simulink model "snap2_f_200msps_64i_4096c_for_8_11_2124fpg"  (R2019a)
settings: Solver=VariableStepDiscrete  StartTime=0.0  StopTime=10.0  RelTol=1e-3
undefined symbols (not defined in the file): datetime, posixtime
libraries: xbsIndex_r4 x17164, casper_library_misc x251, xps_library x14, casper_library_delays x12, casper_library_sources x4
(part 12 of 196)

== system snap2_f_200msps_64i_4096c_for_8_11_2124fpg/cosmic_ray/buffer_and_packetizer ==
  note: should always be zero
  note: Counter number of clock cycles that the board spends in readout state during one readout.
  note: Enable the timer while the board is in readout_state, reset from software.
  note: 4 delays:
      | 1 for OR before buffer address counter
      | 1 for address counter to start after enabled
      | 1 for buffer
      | 1 for block sel
  note: note: buffer address increments 2 clock cycles after read_word
  note: This controls the wait between packets.
  In1 : Inport
  In2 : Inport  Port=2
  In3 : Inport  Port=3
  In4 : Inport  Port=4
  In5 : Inport  Port=5
  In6 : Inport  Port=6
  In7 : Inport  Port=7
  In8 : Inport  Port=8
  In9 : Inport  Port=9
  AddSub : xbsIndex_r4/AddSub  mode=Addition  use_carryin=off  use_carryout=off  en=off  latency=1  precision=Full  arith_type=Unsigned  n_bits=16  bin_pt=14  quantization=Truncate  overflow=Wrap  use_behavioral_HDL=off  hw_selection=Fabric  pipelined=off  dbl_ovrd=off  block_type=addsub
  Constant22 : xbsIndex_r4/Constant  const=0  gui_display_data_type=Boolean  arith_type=Boolean  n_bits=16  bin_pt=14  preci_type=Single  exp_width=8  frac_width=24  explicit_period=on  period=1  equ=P=C  opselect=C  inp2=PCIN>>17  opr=+  inp1=P  carry=CIN  dbl_ovrd=off  block_type=constant
  Constant23 : xbsIndex_r4/Constant  const=0  gui_display_data_type=Boolean  arith_type=Boolean  n_bits=16  bin_pt=14  preci_type=Single  exp_width=8  frac_width=24  explicit_period=on  period=1  equ=P=C  opselect=C  inp2=PCIN>>17  opr=+  inp1=P  carry=CIN  dbl_ovrd=off  block_type=constant
  Constant25 : xbsIndex_r4/Constant  const=3  gui_display_data_type=Fixed-point  arith_type=Unsigned  n_bits=32  bin_pt=0  preci_type=Single  exp_width=8  frac_width=24  explicit_period=on  period=1  equ=P=C  opselect=C  inp2=PCIN>>17  opr=+  inp1=P  carry=CIN  dbl_ovrd=off  block_type=constant
  Constant26 : xbsIndex_r4/Constant  const=2  gui_display_data_type=Fixed-point  arith_type=Unsigned  n_bits=32  bin_pt=0  preci_type=Single  exp_width=8  frac_width=24  explicit_period=on  period=1  equ=P=C  opselect=C  inp2=PCIN>>17  opr=+  inp1=P  carry=CIN  dbl_ovrd=off  block_type=constant
  Constant28 : xbsIndex_r4/Constant  const=0  gui_display_data_type=Boolean  arith_type=Boolean  n_bits=16  bin_pt=14  preci_type=Single  exp_width=8  frac_width=24  explicit_period=on  period=1  equ=P=C  opselect=C  inp2=PCIN>>17  opr=+  inp1=P  carry=CIN  dbl_ovrd=off  block_type=constant
  Constant29 : xbsIndex_r4/Constant  const=1  gui_display_data_type=Fixed-point  arith_type=Unsigned  n_bits=32  bin_pt=0  preci_type=Single  exp_width=8  frac_width=24  explicit_period=on  period=1  equ=P=C  opselect=C  inp2=PCIN>>17  opr=+  inp1=P  carry=CIN  dbl_ovrd=off  block_type=constant
  Constant30 : xbsIndex_r4/Constant  const=0  gui_display_data_type=Fixed-point  arith_type=Unsigned  n_bits=32  bin_pt=0  preci_type=Single  exp_width=8  frac_width=24  explicit_period=on  period=1  equ=P=C  opselect=C  inp2=PCIN>>17  opr=+  inp1=P  carry=CIN  dbl_ovrd=off  block_type=constant
  Constant33 : xbsIndex_r4/Constant  const=256  gui_display_data_type=Fixed-point  arith_type=Unsigned  n_bits=9  bin_pt=0  preci_type=Single  exp_width=8  frac_width=24  explicit_period=on  period=1  equ=P=C  opselect=C  inp2=PCIN>>17  opr=+  inp1=P  carry=CIN  dbl_ovrd=off  block_type=constant
  Counter2 : xbsIndex_r4/Counter  cnt_type=Free Running  cnt_to=Inf  operation=Up  start_count=0  cnt_by_val=1  arith_type=Unsigned  n_bits=32  bin_pt=0  load_pin=off  rst=off  en=on  explicit_period=on  period=1  use_behavioral_HDL=off  implementation=Fabric  dbl_ovrd=off  block_type=counter
  Counter5 : xbsIndex_r4/Counter  cnt_type=Free Running  cnt_to=Inf  operation=Up  start_count=0  cnt_by_val=1  arith_type=Unsigned  n_bits=32  bin_pt=0  load_pin=off  rst=off  en=on  explicit_period=on  period=1  use_behavioral_HDL=off  implementation=Fabric  dbl_ovrd=off  block_type=counter
  Delay1 : xbsIndex_r4/Delay  rst=off  infoeditControl=Selection of Reset will increase slice count due to use of real FFs and instead of SRLs  en=off  latency=2  reg_retiming=off  dbl_ovrd=off  block_type=delay
  Delay{2,4..6,10} (5 alike) : xbsIndex_r4/Delay  rst=off  infoeditControl=Selection of Reset will increase slice count due to use of real FFs and instead of SRLs  en=off  latency=1  reg_retiming=on  dbl_ovrd=off  block_type=delay
  Delay3 : xbsIndex_r4/Delay  rst=off  infoeditControl=Selection of Reset will increase slice count due to use of real FFs and instead of SRLs  en=off  latency=1  reg_retiming=off  dbl_ovrd=off  block_type=delay
  Delay7 : xbsIndex_r4/Delay  rst=off  infoeditControl=Selection of Reset will increase slice count due to use of real FFs and instead of SRLs  en=off  latency=4  reg_retiming=off  dbl_ovrd=off  block_type=delay
  Delay8 : xbsIndex_r4/Delay  rst=off  infoeditControl=Selection of Reset will increase slice count due to use of real FFs and instead of SRLs  en=off  latency=4  reg_retiming=off  dbl_ovrd=off  block_type=delay
  Delay9 : xbsIndex_r4/Delay  rst=off  infoeditControl=Selection of Reset will increase slice count due to use of real FFs and instead of SRLs  en=off  latency=2  reg_retiming=off  dbl_ovrd=off  block_type=delay
  Inverter : xbsIndex_r4/Inverter  en=off  latency=1  dbl_ovrd=off  block_type=inv
  Logical3 : xbsIndex_r4/Logical  logical_function=AND  inputs=2  en=off  latency=1  precision=Full  arith_type=Unsigned  n_bits=16  bin_pt=0  align_bp=on  dbl_ovrd=off  block_type=logical
  Logical4 : xbsIndex_r4/Logical  logical_function=AND  inputs=2  en=off  latency=1  precision=Full  arith_type=Unsigned  n_bits=16  bin_pt=0  align_bp=on  dbl_ovrd=off  block_type=logical
  Logical7 : xbsIndex_r4/Logical  logical_function=OR  inputs=2  en=off  latency=1  precision=Full  arith_type=Unsigned  n_bits=16  bin_pt=0  align_bp=on  dbl_ovrd=off  block_type=logical
  Mux1 : xbsIndex_r4/Mux  inputs=4  en=off  latency=1  precision=Full  arith_type=Unsigned  n_bits=16  bin_pt=14  quantization=Truncate  overflow=Wrap  dbl_ovrd=off  block_type=mux
  Relational2 : xbsIndex_r4/Relational  mode=a>b  op_type=Bool  en=off  latency=1  dbl_ovrd=off  block_type=relational
  Scope{3..5,7..15,18} (13 alike) : Scope
  Single Port RAM{,1..4} (5 alike) : xbsIndex_r4/Single Port RAM  depth=4096  initVector=sin(pi*(0:15)/16)  distributed_mem=Block RAM  write_mode=Read After Write  rst=off  init_reg=0  en=off  latency=1  optimize=Power  dbl_ovrd=off  block_type=spram
  Slice2 : xbsIndex_r4/Slice  nbits=1  boolean_output=on  mode=Upper Bit Location + Width  bit1=0  base1=MSB of Input  bit0=0  base0=LSB of Input  dbl_ovrd=off  block_type=slice
  Slice3 : xbsIndex_r4/Slice  nbits=1  boolean_output=on  mode=Upper Bit Location + Width  bit1=0  base1=MSB of Input  bit0=0  base0=LSB of Input  dbl_ovrd=off  block_type=slice
  Terminator21 : Terminator
  block_counter : xbsIndex_r4/Counter  cnt_type=Free Running  cnt_to=Inf  operation=Up  start_count=0  cnt_by_val=1  arith_type=Unsigned  n_bits=2  bin_pt=0  load_pin=off  rst=off  en=on  explicit_period=on  period=1  use_behavioral_HDL=off  implementation=Fabric  dbl_ovrd=off  block_type=counter
  buffer_addr : xbsIndex_r4/Counter  cnt_type=Free Running  cnt_to=Inf  operation=Up  start_count=0  cnt_by_val=1  arith_type=Unsigned  n_bits=12  bin_pt=0  load_pin=off  rst=off  en=on  explicit_period=on  period=1  use_behavioral_HDL=off  implementation=Fabric  dbl_ovrd=off  block_type=counter
  bus_create : Subsystem  AncestorBlock=casper_library_flow_control/bus_create  mask(bus_create){inputNum=3}
      mask prompts: inputNum "Number of inputs:"
      mask init:
      | bus_create_init(gcb,...
      |     'inputNum', inputNum);
  bus_create1 : Subsystem  AncestorBlock=casper_library_flow_control/bus_create  mask(bus_create){inputNum=3}
      mask prompts: inputNum "Number of inputs:"
      mask init:
      | bus_create_init(gcb,...
      |     'inputNum', inputNum);
  bus_create2 : Subsystem  AncestorBlock=casper_library_flow_control/bus_create  mask(bus_create){inputNum=3}
      mask prompts: inputNum "Number of inputs:"
      mask init:
      | bus_create_init(gcb,...
      |     'inputNum', inputNum);
  bus_create3 : Subsystem  AncestorBlock=casper_library_flow_control/bus_create  mask(bus_create){inputNum=3}
      mask prompts: inputNum "Number of inputs:"
      mask init:
      | bus_create_init(gcb,...
      |     'inputNum', inputNum);
  bus_expand : Subsystem  AncestorBlock=casper_library_flow_control/bus_expand  mask(bus_expand){mode=divisions of equal size, outputNum=4, outputWidth=160, outputBinaryPt=0, outputArithmeticType=0, show_format=off, outputToWorkspace=off, variablePrefix=out, outputToModelAsWell=on}
      mask prompts: outputNum "Number of outputs:"; outputWidth "Output width:"; outputBinaryPt "Output binary point position:"; outputArithmeticType "Output arithmetic type (ufix=0, fix=1, bool=2):"; show_format "Print format string?"; outputToWorkspace "Output to workspace?"; variablePrefix "Variable name prefix:"; outputToModelAsWell "Output to model as well?"
      mask help:
      | Expands a 'bus' made using a bus_create or Xilinx
      | concat block.
      | The input will be divided into the specified number
      | of outputs with the specified arithmetic types.
      | Divisions are made from most significant bit.
      mask init: same as snap2_f_200msps_64i_4096c_for_8_11_2124fpg/autocorr/bus_expand
      settings: AttributesFormatString=4 outputs
      mask TypeOptions mode: divisions of equal size | divisions of arbitrary size
      mask Callback mode: bus_expand_callback();
      mask Callback outputWidth: bus_expand_callback();
      mask Callback outputToWorkspace: bus_expand_callback();
  count-limited-counter : Subsystem
  edge_detect11 : Subsystem  AncestorBlock=casper_library_misc/edge_detect  mask(edge_detect){edge=Falling, polarity=Active High, x_in=[0 0.08 0.08 0.16 0.16 0.24 0.24 0.32 0.32 0.4], y_in=[-1 -1  1  1  1  1  1  1  1  1], x_out=[0.6 0.68 0.68 0.76 0.76 0.84 0.84 0.92 0.92 1], y_out=[-1 -1  1  1 -1 -1 -1 -1 -1 -1]}
      mask prompts: edge "Edge Type"; polarity "Output Polarity"; x_in "Input X Positions"; y_in "Input Y Positions"; x_out "Output X Positions"; y_out "Output Y Positions"
      mask help: Output is active for one-cycle after each edge of the specified type (i.e. rising, falling or both).
      mask init: same as snap2_f_200msps_64i_4096c_for_8_11_2124fpg/pulse_ext/posedge
      settings: AttributesFormatString=|
      | falling edges
      | active high
      mask Help: Output is active for one-cycle after each edge of the specified type (i.e. rising, falling, or both).  Latency is zero.
      mask TypeOptions edge: Rising | Falling | Both
      mask TypeOptions polarity: Active High | Active Low
      mask Display:
      | plot(x_in_num,0.5+y_in_num*0.2);
      | plot(x_out_num,0.5+y_out_num*0.2);
  edge_detect12 : Subsystem  AncestorBlock=casper_library_misc/edge_detect  mask(edge_detect){edge=Falling, polarity=Active High, x_in=[0 0.08 0.08 0.16 0.16 0.24 0.24 0.32 0.32 0.4], y_in=[-1 -1  1  1  1  1  1  1  1  1], x_out=[0.6 0.68 0.68 0.76 0.76 0.84 0.84 0.92 0.92 1], y_out=[-1 -1  1  1 -1 -1 -1 -1 -1 -1]}
      mask prompts: edge "Edge Type"; polarity "Output Polarity"; x_in "Input X Positions"; y_in "Input Y Positions"; x_out "Output X Positions"; y_out "Output Y Positions"
      mask help: Output is active for one-cycle after each edge of the specified type (i.e. rising, falling or both).
      mask init: same as snap2_f_200msps_64i_4096c_for_8_11_2124fpg/pulse_ext/posedge
      settings: AttributesFormatString=|
      | falling edges
      | active high
      mask Help: Output is active for one-cycle after each edge of the specified type (i.e. rising, falling, or both).  Latency is zero.
      mask TypeOptions edge: Rising | Falling | Both
      mask TypeOptions polarity: Active High | Active Low
      mask Display:
      | plot(x_in_num,0.5+y_in_num*0.2);
      | plot(x_out_num,0.5+y_out_num*0.2);
  edge_detect13 : Subsystem  AncestorBlock=casper_library_misc/edge_detect  mask(edge_detect){edge=Falling, polarity=Active High, x_in=[0 0.08 0.08 0.16 0.16 0.24 0.24 0.32 0.32 0.4], y_in=[-1 -1  1  1  1  1  1  1  1  1], x_out=[0.6 0.68 0.68 0.76 0.76 0.84 0.84 0.92 0.92 1], y_out=[-1 -1  1  1 -1 -1 -1 -1 -1 -1]}
      mask prompts: edge "Edge Type"; polarity "Output Polarity"; x_in "Input X Positions"; y_in "Input Y Positions"; x_out "Output X Positions"; y_out "Output Y Positions"
      mask help: Output is active for one-cycle after each edge of the specified type (i.e. rising, falling or both).
      mask init: same as snap2_f_200msps_64i_4096c_for_8_11_2124fpg/pulse_ext/posedge
      settings: AttributesFormatString=|
      | falling edges
      | active high
      mask Help: Output is active for one-cycle after each edge of the specified type (i.e. rising, falling, or both).  Latency is zero.
      mask TypeOptions edge: Rising | Falling | Both
      mask TypeOptions polarity: Active High | Active Low
      mask Display:
      | plot(x_in_num,0.5+y_in_num*0.2);
      | plot(x_out_num,0.5+y_out_num*0.2);
  edge_detect15 : casper_library_misc/edge_detect  edge=Rising  polarity=Active High  x_in=[0 0.08 0.08 0.16 0.16 0.24 0.24 0.32 0.32 0.4]  y_in="[-1 -1  1  1  1  1  1  1  1  1]"  x_out=[0.6 0.68 0.68 0.76 0.76 0.84 0.84 0.92 0.92 1]  y_out="[-1 -1  1  1 -1 -1 -1 -1 -1 -1]"
  packet_counter : xbsIndex_r4/Counter  cnt_type=Free Running  cnt_to=Inf  operation=Up  start_count=0  cnt_by_val=1  arith_type=Unsigned  n_bits=4  bin_pt=0  load_pin=off  rst=off  en=on  explicit_period=on  period=1  use_behavioral_HDL=off  implementation=Fabric  dbl_ovrd=off  block_type=counter
  readout_timer1 : xbsIndex_r4/Counter  cnt_type=Free Running  cnt_to=1  operation=Up  start_count=0  cnt_by_val=1  arith_type=Unsigned  n_bits=32  bin_pt=0  load_pin=off  rst=on  en=on  explicit_period=off  period=1  use_behavioral_HDL=off  implementation=Fabric  dbl_ovrd=off  block_type=counter
  timestamp : xbsIndex_r4/Counter  cnt_type=Free Running  cnt_to=Inf  operation=Up  start_count=0  cnt_by_val=1  arith_type=Unsigned  n_bits=64  bin_pt=0  load_pin=off  rst=on  en=off  explicit_period=on  period=1  use_behavioral_HDL=on  implementation=Fabric  dbl_ovrd=off  block_type=counter
  Out1 : Outport
  Out2 : Outport  Port=2
  Out3 : Outport  Port=3
  Out4 : Outport  Port=4
  Out5 : Outport  Port=5
  Out6 : Outport  Port=6
  Out7 : Outport  Port=7
  Out8 : Outport  Port=8
  Out9 : Outport  Port=9
  Out10 : Outport  Port=10
  Out11 : Outport  Port=11
  Out12 : Outport  Port=12
  Out13 : Outport  Port=13
  timestamp_out : Outport  Port=14
  wires:
  [cr_eof] -> packet_counter/1
  bus_create/1(bus_out) -> Mux1/5
  bus_expand/3(out2) -> Single Port RAM2/2
  timestamp/1 -> Delay2/1, timestamp_out/1
  Logical3/1 -> Counter5/1
  Constant30/1 -> bus_create3/2(in2)
  AddSub/1 -> count-limited-counter/2(limit)
  [read_word] -> Scope3/1
  [cr_40g_dest_ip] -> Out4/1
  Slice3/1 -> edge_detect13/1(in)
  [cr_eof] -> Scope4/1
  [increment_block] -> block_counter/1
  Delay9/1 -> [buffer_we]
  In1/1 -> [packetwait]
  Relational2/1 -> Delay3/1, edge_detect11/1(in)
  Slice2/1 -> edge_detect12/1(in)
  [buffer_we] -> Logical4/2, Scope11/1
  [readout_state] -> Scope8/1
  Constant22/1 -> Out8/1
  bus_expand/4(lsb_out1) -> Single Port RAM3/2
  edge_detect12/1(out) -> [increment_block]
  [buffer_we] -> Single Port RAM1/3
  [packet_subset_count] -> Scope14/1
  Inverter/1 -> [addr_ctr_stop], Delay9/1
  bus_create2/1(bus_out) -> Mux1/3
  Single Port RAM/1 -> bus_create3/1(in1)
  Single Port RAM4/1 -> bus_create2/3(in3), bus_create3/3(in3), bus_create1/3(in3), bus_create/3(in3)
  Delay8/1 -> Out3/1
  [cr_40g_dest_port] -> Out5/1
  Constant23/1 -> Out7/1
  Constant28/1 -> Out9/1
  Logical7/1 -> buffer_addr/1
  [buffer_we] -> Single Port RAM4/3
  In2/1 -> [timestamp_ctr_rst]
  In3/1 -> [packetizer_sync]
  [read_word] -> Logical4/1, Scope5/1
  [increment_block] -> Scope9/1
  packet_counter/1 -> Slice2/1, [packet_subset_count]
  readout_timer1/1 -> Out12/1, Scope15/1
  In5/1 -> [cr_40g_dest_ip]
  [readout_state] -> count-limited-counter/1(en)
  Counter2/1 -> Out10/1
  Mux1/1 -> Out2/1
  bus_expand/1(msb_out4) -> Single Port RAM/2
  [timestamp_ctr_rst] -> timestamp/1
  In4/1 -> [cr_40g_rst]
  [read_word] -> Logical3/2
  In6/1 -> [cr_40g_dest_port]
  In8/1 -> bus_expand/1(bus_in)
  edge_detect15/1 -> readout_timer1/1
  [readout_timer_rst] -> edge_detect15/1
  [packetizer_sync] -> Delay10/1
  [buffer_we] -> Single Port RAM2/3
  bus_create3/1(bus_out) -> Mux1/2
  [cr_40g_rst] -> Out1/1
  Delay7/1 -> Out6/1
  Single Port RAM3/1 -> bus_create/1(in1)
  Logical4/1 -> Scope7/1
  [cr_eof] -> Counter2/1
  [packetwait] -> AddSub/2, Relational2/2
  Single Port RAM2/1 -> bus_create1/1(in1)
  In7/1 -> [readout_timer_rst]
  [addr_ctr_stop] -> Logical7/2
  Constant26/1 -> bus_create1/2(in2)
  [cr_eof] -> Logical3/1
  [buffer_addr] -> Single Port RAM/1
  Constant29/1 -> bus_create2/2(in2)
  [buffer_addr] -> Single Port RAM1/1
  [buffer_we] -> Single Port RAM/3
  Constant33/1 -> AddSub/1
  edge_detect13/1(out) -> [readout_done]
  [cr_eof] -> Delay7/1
  bus_expand/2(out3) -> Single Port RAM1/2
  Delay10/1 -> Delay4/1
  [block_step] -> Scope13/1
  [buffer_addr] -> Single Port RAM4/1
  Delay6/1 -> Terminator21/1
  count-limited-counter/1(out) -> Scope18/1, Relational2/1
  Delay1/1 -> Mux1/1
  Single Port RAM1/1 -> bus_create2/1(in1)
  [readout_state] -> readout_timer1/2
  [buffer_addr] -> Single Port RAM3/1
  [buffer_addr] -> Single Port RAM2/1
  edge_detect11/1(out) -> [cr_eof]
  [block_step] -> Delay1/1
  block_counter/1 -> [block_step], Slice3/1
  [readout_state] -> Inverter/1
  buffer_addr/1 -> [buffer_addr]
  Delay5/1 -> Delay6/1
  Constant25/1 -> bus_create/2(in2)
  Delay3/1 -> [read_word]
  [buffer_we] -> Single Port RAM3/3
  Counter5/1 -> Out11/1
  Delay2/1 -> Single Port RAM4/2
  [read_word] -> Logical7/1
  [buffer_addr] -> Scope12/1
  Delay4/1 -> Delay5/1
  [read_word] -> Delay8/1
  [readout_done] -> Scope10/1
  bus_create1/1(bus_out) -> Mux1/4
  In9/1 -> [readout_state]
  [readout_done] -> Out13/1

== system snap2_f_200msps_64i_4096c_for_8_11_2124fpg/cosmic_ray/bus_create4 ==
  in1 : Inport
  in2 : Inport  Port=2
  concatenate : xbsIndex_r4/Concat  num_inputs=2  dbl_ovrd=off  block_type=concat
  reinterpret1 : xbsIndex_r4/Reinterpret  force_arith_type=on  arith_type=Unsigned  force_bin_pt=on  bin_pt=0  block_type=reinterpret
      settings: infoedit="Changes signal type without altering the binary representation.   You can changed the signal between signed and unsigned, and relocate the binary point.<br><br>Hardware notes: In hardware this block costs nothing.<br><br>Example:  Suppose the input is 6 bits wide, signed, with 2 fractional bits, and the output is forced to unsigned with 0 fractional bits.  Then an input of -2.0 (1110.00 in binary 2's complement) becomes an output of 56 (111000 in binary)."
  reinterpret2 : xbsIndex_r4/Reinterpret  force_arith_type=on  arith_type=Unsigned  force_bin_pt=on  bin_pt=0  block_type=reinterpret
      settings: infoedit="Changes signal type without altering the binary representation.   You can changed the signal between signed and unsigned, and relocate the binary point.<br><br>Hardware notes: In hardware this block costs nothing.<br><br>Example:  Suppose the input is 6 bits wide, signed, with 2 fractional bits, and the output is forced to unsigned with 0 fractional bits.  Then an input of -2.0 (1110.00 in binary 2's complement) becomes an output of 56 (111000 in binary)."
  bus_out : Outport
  wires:
  concatenate/1 -> bus_out/1
  in1/1 -> reinterpret1/1
  reinterpret1/1 -> concatenate/1
  in2/1 -> reinterpret2/1
  reinterpret2/1 -> concatenate/2

== system snap2_f_200msps_64i_4096c_for_8_11_2124fpg/cosmic_ray/bus_create5 ==
  in1 : Inport
  in2 : Inport  Port=2
  concatenate : xbsIndex_r4/Concat  num_inputs=2  dbl_ovrd=off  block_type=concat
  reinterpret1 : xbsIndex_r4/Reinterpret  force_arith_type=on  arith_type=Unsigned  force_bin_pt=on  bin_pt=0  block_type=reinterpret
      settings: infoedit="Changes signal type without altering the binary representation.   You can changed the signal between signed and unsigned, and relocate the binary point.<br><br>Hardware notes: In hardware this block costs nothing.<br><br>Example:  Suppose the input is 6 bits wide, signed, with 2 fractional bits, and the output is forced to unsigned with 0 fractional bits.  Then an input of -2.0 (1110.00 in binary 2's complement) becomes an output of 56 (111000 in binary)."
  reinterpret2 : xbsIndex_r4/Reinterpret  force_arith_type=on  arith_type=Unsigned  force_bin_pt=on  bin_pt=0  block_type=reinterpret
      settings: infoedit="Changes signal type without altering the binary representation.   You can changed the signal between signed and unsigned, and relocate the binary point.<br><br>Hardware notes: In hardware this block costs nothing.<br><br>Example:  Suppose the input is 6 bits wide, signed, with 2 fractional bits, and the output is forced to unsigned with 0 fractional bits.  Then an input of -2.0 (1110.00 in binary 2's complement) becomes an output of 56 (111000 in binary)."
  bus_out : Outport
  wires:
  reinterpret2/1 -> concatenate/2
  in2/1 -> reinterpret2/1
  reinterpret1/1 -> concatenate/1
  in1/1 -> reinterpret1/1
  concatenate/1 -> bus_out/1

== system snap2_f_200msps_64i_4096c_for_8_11_2124fpg/cosmic_ray/coincidence_trigger ==
  note: FIR here?
  note: FIR here?
  note: The ant_threshold block should output a 64 bit signal which is 64 boolean signals bussed together, each indicating whether or not the signal on that antenna exceeded the threshold.
  note: The select_trigger_antennas block sets to zero all streams which are not participating in the core trigger. The select_vetos block sets to zero all antenna streams which are not veto antennas.
  note: Extend pulse for time to wait between trigger and veto.
  note: FIR here?
  note: Determine whether there has been a trigger and not a veto.
  note: FIR here?
  note: Square each voltage stream by copying the bussed voltages and multiplying together, elementwise.
  note: Trigger coincidencer and veto coincidencer are the same block with a different window length.
  In1 : Inport
  In2 : Inport  Port=2
  In3 : Inport  Port=3
  In4 : Inport  Port=4
  In5 : Inport  Port=5
  In6 : Inport  Port=6
  In7 : Inport  Port=7
  In8 : Inport  Port=8
  In9 : Inport  Port=9
  In10 : Inport  Port=10
  AddSub1 : xbsIndex_r4/AddSub  mode=Addition  use_carryin=off  use_carryout=off  en=off  latency=1  precision=Full  arith_type=Unsigned  n_bits=16  bin_pt=14  quantization=Truncate  overflow=Wrap  use_behavioral_HDL=off  hw_selection=Fabric  pipelined=off  dbl_ovrd=off  block_type=addsub
  Convert : xbsIndex_r4/Convert  gui_display_data_type=Boolean  arith_type=Boolean  n_bits=16  bin_pt=14  float_type=Single  exp_bits=8  fraction_bits=24  quantization=Truncate  overflow=Wrap  en=off  latency=1  pipeline=off  dbl_ovrd=off  block_type=convert
  Convert1 : xbsIndex_r4/Convert  gui_display_data_type=Boolean  arith_type=Boolean  n_bits=16  bin_pt=14  float_type=Single  exp_bits=8  fraction_bits=24  quantization=Truncate  overflow=Wrap  en=off  latency=1  pipeline=off  dbl_ovrd=off  block_type=convert
  Delay{10..11,14..20} (9 alike) : xbsIndex_r4/Delay  rst=off  infoeditControl=Selection of Reset will increase slice count due to use of real FFs and instead of SRLs  en=off  latency=1  reg_retiming=off  dbl_ovrd=off  block_type=delay
  Inverter2 : xbsIndex_r4/Inverter  en=off  latency=1  dbl_ovrd=off  block_type=inv
  Logical10 : xbsIndex_r4/Logical  logical_function=AND  inputs=2  en=off  latency=1  precision=Full  arith_type=Unsigned  n_bits=16  bin_pt=0  align_bp=on  dbl_ovrd=off  block_type=logical
  Logical6 : xbsIndex_r4/Logical  logical_function=AND  inputs=2  en=off  latency=1  precision=Full  arith_type=Unsigned  n_bits=16  bin_pt=0  align_bp=on  dbl_ovrd=off  block_type=logical
  Logical9 : xbsIndex_r4/Logical  logical_function=AND  inputs=2  en=off  latency=1  precision=Full  arith_type=Unsigned  n_bits=16  bin_pt=0  align_bp=on  dbl_ovrd=off  block_type=logical
  Relational3 : xbsIndex_r4/Relational  mode=a>b  op_type=Bool  en=off  latency=1  dbl_ovrd=off  block_type=relational
  Relational4 : xbsIndex_r4/Relational  mode=a>b  op_type=Bool  en=off  latency=1  dbl_ovrd=off  block_type=relational
  Scope{17,19..23,25..30} (12 alike) : Scope
  bus_threshold 1 : Subsystem
  bus_threshold 2 : Subsystem
  custom_pulse_extend_with_sync : Subsystem
  custom_pulse_extend_with_sync1 : Subsystem
  divide_by_2 : xbsIndex_r4/Shift  shift_dir=Left  shift_bits=1  en=off  latency=1  precision=Full  arith_type="Signed  (2's comp)"  n_bits=16  bin_pt=14  quantization=Truncate  overflow=Wrap  dbl_ovrd=off  block_type=shift
  square_bus_components : Subsystem
  trigger_coincidencer : Subsystem
  veto_coincidencer : Subsystem
  trigger : Outport
  core_coincidence : Outport  Port=2
  veto_detected : Outport  Port=3
  wires:
  bus_threshold 2/2(sync_out) -> Delay20/1
  In10/1 -> [veto_antennas]
  Delay11/1 -> custom_pulse_extend_with_sync/3(sync_in)
  bus_threshold 2/1(OUT) -> Logical10/2
  [veto_ant_thresh] -> bus_threshold 2/1(threshold)
  [coinc_window] -> trigger_coincidencer/1(window_length)
  In9/1 -> [trigger_antennas]
  Delay19/1 -> trigger_coincidencer/3(sync_in)
  custom_pulse_extend_with_sync1/1("out ") -> Scope22/1, Inverter2/1
  In4/1 -> [veto_ant_thresh]
  [veto_window] -> veto_coincidencer/1(window_length)
  [coinc_thresh] -> Relational4/2
  [window_average] -> custom_pulse_extend_with_sync1/2("pulse_length ")
  In1/1 -> square_bus_components/1(IN)
  bus_threshold 1/2(sync_out) -> Delay19/1
  Logical10/1 -> Scope19/1, veto_coincidencer/2(data_bus_in)
  Logical6/1 -> trigger/1
  Delay20/1 -> veto_coincidencer/3(sync_in)
  Inverter2/1 -> Scope26/1, Logical6/2
  Logical9/1 -> Scope20/1, trigger_coincidencer/2(data_bus_in)
  Delay18/1 -> Scope23/1
  In7/1 -> [coinc_thresh]
  custom_pulse_extend_with_sync1/2(sync_out) -> Convert1/1, Delay14/1
  In8/1 -> [veto_thresh]
  trigger_coincidencer/2("out ") -> Scope27/1, Relational4/1
  Delay17/1 -> Scope17/1
  [trigger_antennas] -> Logical9/1
  [coinc_window] -> AddSub1/2
  [veto_window] -> AddSub1/1
  veto_coincidencer/2("out ") -> Relational3/1, Scope28/1
  In2/1 -> [sync]
  Delay14/1 -> Delay18/1
  veto_coincidencer/1(sync_out) -> Delay10/1
  custom_pulse_extend_with_sync/2(sync_out) -> Delay16/1
  AddSub1/1 -> divide_by_2/1
  Relational3/1 -> Scope30/1, custom_pulse_extend_with_sync1/1("in ")
  In5/1 -> [coinc_window]
  In3/1 -> [ant_thresh]
  [veto_thresh] -> Relational3/2
  Delay16/1 -> Delay17/1
  Relational4/1 -> Scope29/1, custom_pulse_extend_with_sync/1("in ")
  [window_average] -> custom_pulse_extend_with_sync/2("pulse_length ")
  square_bus_components/2(sync_out) -> bus_threshold 2/3(sync_in), bus_threshold 1/3(sync_in)
  divide_by_2/1 -> [window_average]
  [ant_thresh] -> bus_threshold 1/1(threshold)
  square_bus_components/1(OUT) -> bus_threshold 1/2(bus_in), bus_threshold 2/2(bus_in)
  Delay15/1 -> Scope25/1, Logical6/1
  trigger_coincidencer/1(sync_out) -> Delay11/1
  [sync] -> square_bus_components/2(sync_in)
  [veto_antennas] -> Logical10/1
  In6/1 -> [veto_window]
  Delay10/1 -> custom_pulse_extend_with_sync1/3(sync_in)
  bus_threshold 1/1(OUT) -> Logical9/2
  custom_pulse_extend_with_sync/1("out ") -> Delay15/1, Scope21/1, Convert/1
  Convert/1 -> core_coincidence/1
  Convert1/1 -> veto_detected/1
